simulink model "fftwbr_core"  (Simulink 7.9)
settings: Solver=ode45  StartTime=0.0  StopTime=10.0  RelTol=1e-3
libraries: xbsIndex_r4 x15792, casper_library_misc x464, casper_library_delays x57, xbsReplacements_r4 x8, casper_library_ffts_twiddle x4
(part 141 of 233)

== system fftwbr_core/fft_wideband_real1/fft_direct/butterfly4_7/twiddle_general_dsp48e/coeff_gen ==
  rst : Inport
  Counter : xbsIndex_r4/Counter  cnt_type=Free Running  cnt_to=Inf  operation=Up  start_count=0  cnt_by_val=1  arith_type=Unsigned  n_bits=11  bin_pt=0  load_pin=off  rst=on  en=off  explicit_period=on  period=1  dbl_ovrd=off  use_behavioral_HDL=off  implementation=Fabric  block_type=counter
  ROM : xbsIndex_r4/ROM  depth=2048  initVector="[-0.9238739013671875                       -0.9239501953125                    -0.9240264892578125                     -0.924102783203125                    -0.9241790771484375                    -0.9242401123046875                         -0.92431640625                    -0.9243927001953125                     -0.924468994140625                    -0.9245452880859375                    -0.9246063232421875                       -0.9246826171875                    -0.9247589111328125                     -0.924835205078125                     -0.924896240234375                    -0.9249725341796875                        -0.925048828125                    -0.9251251220703125                     -0.925201416015625                     -0.925262451171875                    -0.9253387451171875                       -0.9254150390625                    -0.9254913330078125                    -0.9255523681640625                     -0.925628662109375                    -0.9257049560546875                            -0.92578125                      -0.92584228515625                    -0.9259185791015625                     -0.925994873046875                    -0.9260711669921875                    -0.9261322021484375                      -0.92620849609375                    -0.9262847900390625                     -0.926361083984375                     -0.926422119140625                    -0.9264984130859375                      -0.92657470703125                       -0.9266357421875                    -0.9267120361328125                     -0.926788330078125                    -0.9268646240234375                    -0.9269256591796875                        -0.927001953125                    -0.9270782470703125                     -0.927154541015625                     -0.927215576171875                    -0.9272918701171875                       -0.9273681640625                      -0.92742919921875                    -0.9275054931640625                     -0.927581787109375                     -0.927642822265625                    -0.9277191162109375                      -0.92779541015625                       -0.9278564453125                    -0.9279327392578125                     -0.928009033203125                    -0.9280853271484375                    -0.9281463623046875                         -0.92822265625                    -0.9282989501953125                    -0.9283599853515625                     -0.928436279296875                    -0.9285125732421875                    -0.9285736083984375                      -0.92864990234375                    -0.9287261962890625                    -0.9287872314453125                     -0.928863525390625                    -0.9289398193359375                    -0.9290008544921875                       -0.9290771484375                      -0.92913818359375                    -0.9292144775390625                     -0.929290771484375                     -0.929351806640625                    -0.9294281005859375                      -0.92950439453125                       -0.9295654296875                    -0.9296417236328125                     -0.929718017578125                     -0.929779052734375                    -0.9298553466796875                    -0.9299163818359375                      -0.92999267578125                    -0.9300689697265625                    -0.9301300048828125                     -0.930206298828125                    -0.9302825927734375                    -0.9303436279296875                        -0.930419921875                      -0.93048095703125                    -0.9305572509765625                     -0.930633544921875                     -0.930694580078125                    -0.9307708740234375                    -0.9308319091796875                        -0.930908203125                    -0.9309844970703125                    -0.9310455322265625                     -0.931121826171875                     -0.931182861328125 ...(+75854 chars)]"  distributed_mem=Block RAM  rst=off  init_reg=0  en=off  latency=2  gui_display_data_type=Fixed-point  arith_type="Signed  (2's comp)"  n_bits=18  bin_pt=16  preci_type=Single  exp_width=8  frac_width=24  dbl_ovrd=off  optimize=Area  use_rpm=on  block_type=sprom
  ROM1 : xbsIndex_r4/ROM  depth=2048  initVector="[-0.3826904296875                      -0.38250732421875                         -0.38232421875                    -0.3821563720703125                    -0.3819732666015625                    -0.3817901611328125                     -0.381622314453125                     -0.381439208984375                    -0.3812713623046875                    -0.3810882568359375                    -0.3809051513671875                       -0.3807373046875                      -0.38055419921875                    -0.3803863525390625                    -0.3802032470703125                    -0.3800201416015625                     -0.379852294921875                     -0.379669189453125                     -0.379486083984375                    -0.3793182373046875                    -0.3791351318359375                      -0.37896728515625                       -0.3787841796875                      -0.37860107421875                    -0.3784332275390625                    -0.3782501220703125                    -0.3780670166015625                     -0.377899169921875                     -0.377716064453125                     -0.377532958984375                    -0.3773651123046875                    -0.3771820068359375                      -0.37701416015625                       -0.3768310546875                      -0.37664794921875                    -0.3764801025390625                    -0.3762969970703125                    -0.3761138916015625                     -0.375946044921875                     -0.375762939453125                     -0.375579833984375                    -0.3754119873046875                    -0.3752288818359375                    -0.3750457763671875                       -0.3748779296875                      -0.37469482421875                    -0.3745269775390625                    -0.3743438720703125                    -0.3741607666015625                     -0.373992919921875                     -0.373809814453125                     -0.373626708984375                    -0.3734588623046875                    -0.3732757568359375                    -0.3730926513671875                       -0.3729248046875                      -0.37274169921875                         -0.37255859375                    -0.3723907470703125                    -0.3722076416015625                    -0.3720245361328125                     -0.371856689453125                     -0.371673583984375                     -0.371490478515625                    -0.3713226318359375                    -0.3711395263671875                    -0.3709564208984375                      -0.37078857421875                         -0.37060546875                      -0.37042236328125                    -0.3702545166015625                    -0.3700714111328125                    -0.3698883056640625                     -0.369720458984375                     -0.369537353515625                     -0.369354248046875                    -0.3691864013671875                    -0.3690032958984375                    -0.3688201904296875                         -0.36865234375                      -0.36846923828125                       -0.3682861328125                      -0.36810302734375                    -0.3679351806640625                    -0.3677520751953125                    -0.3675689697265625                     -0.367401123046875                     -0.367218017578125                     -0.367034912109375                    -0.3668670654296875                    -0.3666839599609375                    -0.3665008544921875                       -0.3663330078125                      -0.36614990234375                        -0.365966796875                    -0.3657989501953125                    -0.3656158447265625                    -0.3654327392578125                    -0.3652496337890625                     -0.365081787109375                     -0.364898681640625                     -0.364715576171875                    -0.3645477294921875 ...(+75851 chars)]"  distributed_mem=Block RAM  rst=off  init_reg=0  en=off  latency=2  gui_display_data_type=Fixed-point  arith_type="Signed  (2's comp)"  n_bits=18  bin_pt=16  preci_type=Single  exp_width=8  frac_width=24  dbl_ovrd=off  optimize=Area  use_rpm=on  block_type=sprom
  Slice : xbsIndex_r4/Slice  nbits=11  boolean_output=off  mode=Upper Bit Location + Width  bit1=0  base1=MSB of Input  bit0=0  base0=LSB of Input  dbl_ovrd=off  block_type=slice
  ri_to_c : casper_library_misc/ri_to_c
  w : Outport
  wires:
  ri_to_c/1 -> w/1
  rst/1 -> Counter/1
  Counter/1 -> Slice/1
  Slice/1 -> ROM/1, ROM1/1
  ROM/1 -> ri_to_c/1
  ROM1/1 -> ri_to_c/2
